simulink model "CCMHfaMotor"  (R2022b) (continued)
== system CCMHfaMotor == (continued)
  HfaActr05UrgcSts_HfaActr05UrgcSts/1 -> HfaMotor_Runnable_sys/171(HfaActr05UrgcSts_HfaActr05UrgcSts_read)
  HfaActr05VoltErr_HfaActr05VoltErr/1 -> HfaMotor_Runnable_sys/172(HfaActr05VoltErr_HfaActr05VoltErr_read)
  HfaActr06ActPson_HfaActr06ActPson/1 -> HfaMotor_Runnable_sys/173(HfaActr06ActPson_HfaActr06ActPson_read)
  HfaActr06ActPson_HfaActr06ActPson_IsUpdated/1 -> HfaMotor_Runnable_sys/174(HfaActr06ActPson_HfaActr06ActPson_IsUpdated_read)
  HfaActr06BlckInd_HfaActr06BlckInd/1 -> HfaMotor_Runnable_sys/175(HfaActr06BlckInd_HfaActr06BlckInd_read)
  HfaActr06BlckSts_HfaActr06BlckSts/1 -> HfaMotor_Runnable_sys/176(HfaActr06BlckSts_HfaActr06BlckSts_read)
  HfaActr06BusAdr_HfaActr06BusAdr/1 -> HfaMotor_Runnable_sys/177(HfaActr06BusAdr_HfaActr06BusAdr_read)
  HfaActr06CoilSts_HfaActr06CoilSts/1 -> HfaMotor_Runnable_sys/178(HfaActr06CoilSts_HfaActr06CoilSts_read)
  HfaActr06DrvDir_HfaActr06DrvDir/1 -> HfaMotor_Runnable_sys/179(HfaActr06DrvDir_HfaActr06DrvDir_read)
  HfaActr06ElecErr_HfaActr06ElecErr/1 -> HfaMotor_Runnable_sys/180(HfaActr06ElecErr_HfaActr06ElecErr_read)
  HfaActr06Mode_HfaActr06Mode/1 -> HfaMotor_Runnable_sys/181(HfaActr06Mode_HfaActr06Mode_read)
  HfaActr06MovDir_HfaActr06MovDir/1 -> HfaMotor_Runnable_sys/182(HfaActr06MovDir_HfaActr06MovDir_read)
  HfaActr06OvrTempErr_HfaActr06OvrTempErr/1 -> HfaMotor_Runnable_sys/183(HfaActr06OvrTempErr_HfaActr06OvrTempErr_read)
  HfaActr06PsonSts_HfaActr06PsonSts/1 -> HfaMotor_Runnable_sys/184(HfaActr06PsonSts_HfaActr06PsonSts_read)
  HfaActr06ResetSts_HfaActr06ResetSts/1 -> HfaMotor_Runnable_sys/185(HfaActr06ResetSts_HfaActr06ResetSts_read)
  HfaActr06SpclFct_HfaActr06SpclFct/1 -> HfaMotor_Runnable_sys/186(HfaActr06SpclFct_HfaActr06SpclFct_read)
  HfaActr06SpdSts_HfaActr06SpdSts/1 -> HfaMotor_Runnable_sys/187(HfaActr06SpdSts_HfaActr06SpdSts_read)
  HfaActr06TrqSts_HfaActr06TrqSts/1 -> HfaMotor_Runnable_sys/188(HfaActr06TrqSts_HfaActr06TrqSts_read)
  HfaActr06UrgcExist_HfaActr06UrgcExist/1 -> HfaMotor_Runnable_sys/189(HfaActr06UrgcExist_HfaActr06UrgcExist_read)
  HfaActr06UrgcPson_HfaActr06UrgcPson/1 -> HfaMotor_Runnable_sys/190(HfaActr06UrgcPson_HfaActr06UrgcPson_read)
  HfaActr06UrgcSts_HfaActr06UrgcSts/1 -> HfaMotor_Runnable_sys/191(HfaActr06UrgcSts_HfaActr06UrgcSts_read)
  HfaActr06VoltErr_HfaActr06VoltErr/1 -> HfaMotor_Runnable_sys/192(HfaActr06VoltErr_HfaActr06VoltErr_read)
  HfaActr07ActPson_HfaActr07ActPson/1 -> HfaMotor_Runnable_sys/193(HfaActr07ActPson_HfaActr07ActPson_read)
  HfaActr07ActPson_HfaActr07ActPson_IsUpdated/1 -> HfaMotor_Runnable_sys/194(HfaActr07ActPson_HfaActr07ActPson_IsUpdated_read)
  HfaActr07BlckInd_HfaActr07BlckInd/1 -> HfaMotor_Runnable_sys/195(HfaActr07BlckInd_HfaActr07BlckInd_read)
  HfaActr07BlckSts_HfaActr07BlckSts/1 -> HfaMotor_Runnable_sys/196(HfaActr07BlckSts_HfaActr07BlckSts_read)
  HfaActr07BusAdr_HfaActr07BusAdr/1 -> HfaMotor_Runnable_sys/197(HfaActr07BusAdr_HfaActr07BusAdr_read)
  HfaActr07CoilSts_HfaActr07CoilSts/1 -> HfaMotor_Runnable_sys/198(HfaActr07CoilSts_HfaActr07CoilSts_read)
  HfaActr07DrvDir_HfaActr07DrvDir/1 -> HfaMotor_Runnable_sys/199(HfaActr07DrvDir_HfaActr07DrvDir_read)
  HfaActr07ElecErr_HfaActr07ElecErr/1 -> HfaMotor_Runnable_sys/200(HfaActr07ElecErr_HfaActr07ElecErr_read)
  HfaActr07Mode_HfaActr07Mode/1 -> HfaMotor_Runnable_sys/201(HfaActr07Mode_HfaActr07Mode_read)
  HfaActr07MovDir_HfaActr07MovDir/1 -> HfaMotor_Runnable_sys/202(HfaActr07MovDir_HfaActr07MovDir_read)
  HfaActr07OvrTempErr_HfaActr07OvrTempErr/1 -> HfaMotor_Runnable_sys/203(HfaActr07OvrTempErr_HfaActr07OvrTempErr_read)
  HfaActr07PsonSts_HfaActr07PsonSts/1 -> HfaMotor_Runnable_sys/204(HfaActr07PsonSts_HfaActr07PsonSts_read)
  HfaActr07ResetSts_HfaActr07ResetSts/1 -> HfaMotor_Runnable_sys/205(HfaActr07ResetSts_HfaActr07ResetSts_read)
  HfaActr07SpclFct_HfaActr07SpclFct/1 -> HfaMotor_Runnable_sys/206(HfaActr07SpclFct_HfaActr07SpclFct_read)
  HfaActr07SpdSts_HfaActr07SpdSts/1 -> HfaMotor_Runnable_sys/207(HfaActr07SpdSts_HfaActr07SpdSts_read)
  HfaActr07TrqSts_HfaActr07TrqSts/1 -> HfaMotor_Runnable_sys/208(HfaActr07TrqSts_HfaActr07TrqSts_read)
  HfaActr07UrgcExist_HfaActr07UrgcExist/1 -> HfaMotor_Runnable_sys/209(HfaActr07UrgcExist_HfaActr07UrgcExist_read)
  HfaActr07UrgcPson_HfaActr07UrgcPson/1 -> HfaMotor_Runnable_sys/210(HfaActr07UrgcPson_HfaActr07UrgcPson_read)
  HfaActr07UrgcSts_HfaActr07UrgcSts/1 -> HfaMotor_Runnable_sys/211(HfaActr07UrgcSts_HfaActr07UrgcSts_read)
  HfaActr07VoltErr_HfaActr07VoltErr/1 -> HfaMotor_Runnable_sys/212(HfaActr07VoltErr_HfaActr07VoltErr_read)
  HfaActr08ActPson_HfaActr08ActPson/1 -> HfaMotor_Runnable_sys/213(HfaActr08ActPson_HfaActr08ActPson_read)
  HfaActr08ActPson_HfaActr08ActPson_IsUpdated/1 -> HfaMotor_Runnable_sys/214(HfaActr08ActPson_HfaActr08ActPson_IsUpdated_read)
  HfaActr08BlckInd_HfaActr08BlckInd/1 -> HfaMotor_Runnable_sys/215(HfaActr08BlckInd_HfaActr08BlckInd_read)
  HfaActr08BlckSts_HfaActr08BlckSts/1 -> HfaMotor_Runnable_sys/216(HfaActr08BlckSts_HfaActr08BlckSts_read)
  HfaActr08BusAdr_HfaActr08BusAdr/1 -> HfaMotor_Runnable_sys/217(HfaActr08BusAdr_HfaActr08BusAdr_read)
  HfaActr08CoilSts_HfaActr08CoilSts/1 -> HfaMotor_Runnable_sys/218(HfaActr08CoilSts_HfaActr08CoilSts_read)
  HfaActr08DrvDir_HfaActr08DrvDir/1 -> HfaMotor_Runnable_sys/219(HfaActr08DrvDir_HfaActr08DrvDir_read)
  HfaActr08ElecErr_HfaActr08ElecErr/1 -> HfaMotor_Runnable_sys/220(HfaActr08ElecErr_HfaActr08ElecErr_read)
  HfaActr08Mode_HfaActr08Mode/1 -> HfaMotor_Runnable_sys/221(HfaActr08Mode_HfaActr08Mode_read)
  HfaActr08MovDir_HfaActr08MovDir/1 -> HfaMotor_Runnable_sys/222(HfaActr08MovDir_HfaActr08MovDir_read)
  HfaActr08OvrTempErr_HfaActr08OvrTempErr/1 -> HfaMotor_Runnable_sys/223(HfaActr08OvrTempErr_HfaActr08OvrTempErr_read)
  HfaActr08PsonSts_HfaActr08PsonSts/1 -> HfaMotor_Runnable_sys/224(HfaActr08PsonSts_HfaActr08PsonSts_read)
  HfaActr08ResetSts_HfaActr08ResetSts/1 -> HfaMotor_Runnable_sys/225(HfaActr08ResetSts_HfaActr08ResetSts_read)
  HfaActr08SpclFct_HfaActr08SpclFct/1 -> HfaMotor_Runnable_sys/226(HfaActr08SpclFct_HfaActr08SpclFct_read)
  HfaActr08SpdSts_HfaActr08SpdSts/1 -> HfaMotor_Runnable_sys/227(HfaActr08SpdSts_HfaActr08SpdSts_read)
  HfaActr08TrqSts_HfaActr08TrqSts/1 -> HfaMotor_Runnable_sys/228(HfaActr08TrqSts_HfaActr08TrqSts_read)
  HfaActr08UrgcExist_HfaActr08UrgcExist/1 -> HfaMotor_Runnable_sys/229(HfaActr08UrgcExist_HfaActr08UrgcExist_read)
  HfaActr08UrgcPson_HfaActr08UrgcPson/1 -> HfaMotor_Runnable_sys/230(HfaActr08UrgcPson_HfaActr08UrgcPson_read)
  HfaActr08UrgcSts_HfaActr08UrgcSts/1 -> HfaMotor_Runnable_sys/231(HfaActr08UrgcSts_HfaActr08UrgcSts_read)
  HfaActr08VoltErr_HfaActr08VoltErr/1 -> HfaMotor_Runnable_sys/232(HfaActr08VoltErr_HfaActr08VoltErr_read)
  HfaActr09ActPson_HfaActr09ActPson/1 -> HfaMotor_Runnable_sys/233(HfaActr09ActPson_HfaActr09ActPson_read)
  HfaActr09ActPson_HfaActr09ActPson_IsUpdated/1 -> HfaMotor_Runnable_sys/234(HfaActr09ActPson_HfaActr09ActPson_IsUpdated_read)
  HfaActr09BlckInd_HfaActr09BlckInd/1 -> HfaMotor_Runnable_sys/235(HfaActr09BlckInd_HfaActr09BlckInd_read)
  HfaActr09BlckSts_HfaActr09BlckSts/1 -> HfaMotor_Runnable_sys/236(HfaActr09BlckSts_HfaActr09BlckSts_read)
  HfaActr09BusAdr_HfaActr09BusAdr/1 -> HfaMotor_Runnable_sys/237(HfaActr09BusAdr_HfaActr09BusAdr_read)
  HfaActr09CoilSts_HfaActr09CoilSts/1 -> HfaMotor_Runnable_sys/238(HfaActr09CoilSts_HfaActr09CoilSts_read)
  HfaActr09DrvDir_HfaActr09DrvDir/1 -> HfaMotor_Runnable_sys/239(HfaActr09DrvDir_HfaActr09DrvDir_read)
  HfaActr09ElecErr_HfaActr09ElecErr/1 -> HfaMotor_Runnable_sys/240(HfaActr09ElecErr_HfaActr09ElecErr_read)
  HfaActr09Mode_HfaActr09Mode/1 -> HfaMotor_Runnable_sys/241(HfaActr09Mode_HfaActr09Mode_read)
  HfaActr09MovDir_HfaActr09MovDir/1 -> HfaMotor_Runnable_sys/242(HfaActr09MovDir_HfaActr09MovDir_read)
  HfaActr09OvrTempErr_HfaActr09OvrTempErr/1 -> HfaMotor_Runnable_sys/243(HfaActr09OvrTempErr_HfaActr09OvrTempErr_read)
  HfaActr09PsonSts_HfaActr09PsonSts/1 -> HfaMotor_Runnable_sys/244(HfaActr09PsonSts_HfaActr09PsonSts_read)
  HfaActr09ResetSts_HfaActr09ResetSts/1 -> HfaMotor_Runnable_sys/245(HfaActr09ResetSts_HfaActr09ResetSts_read)
  HfaActr09SpclFct_HfaActr09SpclFct/1 -> HfaMotor_Runnable_sys/246(HfaActr09SpclFct_HfaActr09SpclFct_read)
  HfaActr09SpdSts_HfaActr09SpdSts/1 -> HfaMotor_Runnable_sys/247(HfaActr09SpdSts_HfaActr09SpdSts_read)
  HfaActr09TrqSts_HfaActr09TrqSts/1 -> HfaMotor_Runnable_sys/248(HfaActr09TrqSts_HfaActr09TrqSts_read)
  HfaActr09UrgcExist_HfaActr09UrgcExist/1 -> HfaMotor_Runnable_sys/249(HfaActr09UrgcExist_HfaActr09UrgcExist_read)
  HfaActr09UrgcPson_HfaActr09UrgcPson/1 -> HfaMotor_Runnable_sys/250(HfaActr09UrgcPson_HfaActr09UrgcPson_read)
  HfaActr09UrgcSts_HfaActr09UrgcSts/1 -> HfaMotor_Runnable_sys/251(HfaActr09UrgcSts_HfaActr09UrgcSts_read)
  HfaActr09VoltErr_HfaActr09VoltErr/1 -> HfaMotor_Runnable_sys/252(HfaActr09VoltErr_HfaActr09VoltErr_read)
  HfaActr10ActPson_HfaActr10ActPson/1 -> HfaMotor_Runnable_sys/253(HfaActr10ActPson_HfaActr10ActPson_read)
  HfaActr10ActPson_HfaActr10ActPson_IsUpdated/1 -> HfaMotor_Runnable_sys/254(HfaActr10ActPson_HfaActr10ActPson_IsUpdated_read)
  HfaActr10BlckInd_HfaActr10BlckInd/1 -> HfaMotor_Runnable_sys/255(HfaActr10BlckInd_HfaActr10BlckInd_read)
  HfaActr10BlckSts_HfaActr10BlckSts/1 -> HfaMotor_Runnable_sys/256(HfaActr10BlckSts_HfaActr10BlckSts_read)
  HfaActr10BusAdr_HfaActr10BusAdr/1 -> HfaMotor_Runnable_sys/257(HfaActr10BusAdr_HfaActr10BusAdr_read)
  HfaActr10CoilSts_HfaActr10CoilSts/1 -> HfaMotor_Runnable_sys/258(HfaActr10CoilSts_HfaActr10CoilSts_read)
  HfaActr10DrvDir_HfaActr10DrvDir/1 -> HfaMotor_Runnable_sys/259(HfaActr10DrvDir_HfaActr10DrvDir_read)
  HfaActr10ElecErr_HfaActr10ElecErr/1 -> HfaMotor_Runnable_sys/260(HfaActr10ElecErr_HfaActr10ElecErr_read)
  HfaActr10Mode_HfaActr10Mode/1 -> HfaMotor_Runnable_sys/261(HfaActr10Mode_HfaActr10Mode_read)
  HfaActr10MovDir_HfaActr10MovDir/1 -> HfaMotor_Runnable_sys/262(HfaActr10MovDir_HfaActr10MovDir_read)
  HfaActr10OvrTempErr_HfaActr10OvrTempErr/1 -> HfaMotor_Runnable_sys/263(HfaActr10OvrTempErr_HfaActr10OvrTempErr_read)
  HfaActr10PsonSts_HfaActr10PsonSts/1 -> HfaMotor_Runnable_sys/264(HfaActr10PsonSts_HfaActr10PsonSts_read)
  HfaActr10ResetSts_HfaActr10ResetSts/1 -> HfaMotor_Runnable_sys/265(HfaActr10ResetSts_HfaActr10ResetSts_read)
  HfaActr10SpclFct_HfaActr10SpclFct/1 -> HfaMotor_Runnable_sys/266(HfaActr10SpclFct_HfaActr10SpclFct_read)
  HfaActr10SpdSts_HfaActr10SpdSts/1 -> HfaMotor_Runnable_sys/267(HfaActr10SpdSts_HfaActr10SpdSts_read)
  HfaActr10TrqSts_HfaActr10TrqSts/1 -> HfaMotor_Runnable_sys/268(HfaActr10TrqSts_HfaActr10TrqSts_read)
  HfaActr10UrgcExist_HfaActr10UrgcExist/1 -> HfaMotor_Runnable_sys/269(HfaActr10UrgcExist_HfaActr10UrgcExist_read)
  HfaActr10UrgcPson_HfaActr10UrgcPson/1 -> HfaMotor_Runnable_sys/270(HfaActr10UrgcPson_HfaActr10UrgcPson_read)
  HfaActr10UrgcSts_HfaActr10UrgcSts/1 -> HfaMotor_Runnable_sys/271(HfaActr10UrgcSts_HfaActr10UrgcSts_read)
  HfaActr10VoltErr_HfaActr10VoltErr/1 -> HfaMotor_Runnable_sys/272(HfaActr10VoltErr_HfaActr10VoltErr_read)
  HfaActr11ActPson_HfaActr11ActPson/1 -> HfaMotor_Runnable_sys/273(HfaActr11ActPson_HfaActr11ActPson_read)
  HfaActr11ActPson_HfaActr11ActPson_IsUpdated/1 -> HfaMotor_Runnable_sys/274(HfaActr11ActPson_HfaActr11ActPson_IsUpdated_read)
  HfaActr11BlckInd_HfaActr11BlckInd/1 -> HfaMotor_Runnable_sys/275(HfaActr11BlckInd_HfaActr11BlckInd_read)
  HfaActr11BlckSts_HfaActr11BlckSts/1 -> HfaMotor_Runnable_sys/276(HfaActr11BlckSts_HfaActr11BlckSts_read)
  HfaActr11BusAdr_HfaActr11BusAdr/1 -> HfaMotor_Runnable_sys/277(HfaActr11BusAdr_HfaActr11BusAdr_read)
  HfaActr11CoilSts_HfaActr11CoilSts/1 -> HfaMotor_Runnable_sys/278(HfaActr11CoilSts_HfaActr11CoilSts_read)
  HfaActr11DrvDir_HfaActr11DrvDir/1 -> HfaMotor_Runnable_sys/279(HfaActr11DrvDir_HfaActr11DrvDir_read)
  HfaActr11ElecErr_HfaActr11ElecErr/1 -> HfaMotor_Runnable_sys/280(HfaActr11ElecErr_HfaActr11ElecErr_read)
  HfaActr11Mode_HfaActr11Mode/1 -> HfaMotor_Runnable_sys/281(HfaActr11Mode_HfaActr11Mode_read)
  HfaActr11MovDir_HfaActr11MovDir/1 -> HfaMotor_Runnable_sys/282(HfaActr11MovDir_HfaActr11MovDir_read)
  HfaActr11OvrTempErr_HfaActr11OvrTempErr/1 -> HfaMotor_Runnable_sys/283(HfaActr11OvrTempErr_HfaActr11OvrTempErr_read)
  HfaActr11PsonSts_HfaActr11PsonSts/1 -> HfaMotor_Runnable_sys/284(HfaActr11PsonSts_HfaActr11PsonSts_read)
  HfaActr11ResetSts_HfaActr11ResetSts/1 -> HfaMotor_Runnable_sys/285(HfaActr11ResetSts_HfaActr11ResetSts_read)
  HfaActr11SpclFct_HfaActr11SpclFct/1 -> HfaMotor_Runnable_sys/286(HfaActr11SpclFct_HfaActr11SpclFct_read)
  HfaActr11SpdSts_HfaActr11SpdSts/1 -> HfaMotor_Runnable_sys/287(HfaActr11SpdSts_HfaActr11SpdSts_read)
  HfaActr11TrqSts_HfaActr11TrqSts/1 -> HfaMotor_Runnable_sys/288(HfaActr11TrqSts_HfaActr11TrqSts_read)
  HfaActr11UrgcExist_HfaActr11UrgcExist/1 -> HfaMotor_Runnable_sys/289(HfaActr11UrgcExist_HfaActr11UrgcExist_read)
  HfaActr11UrgcPson_HfaActr11UrgcPson/1 -> HfaMotor_Runnable_sys/290(HfaActr11UrgcPson_HfaActr11UrgcPson_read)
  HfaActr11UrgcSts_HfaActr11UrgcSts/1 -> HfaMotor_Runnable_sys/291(HfaActr11UrgcSts_HfaActr11UrgcSts_read)
  HfaActr11VoltErr_HfaActr11VoltErr/1 -> HfaMotor_Runnable_sys/292(HfaActr11VoltErr_HfaActr11VoltErr_read)
  HfaActr12ActPson_HfaActr12ActPson/1 -> HfaMotor_Runnable_sys/293(HfaActr12ActPson_HfaActr12ActPson_read)
  HfaActr12ActPson_HfaActr12ActPson_IsUpdated/1 -> HfaMotor_Runnable_sys/294(HfaActr12ActPson_HfaActr12ActPson_IsUpdated_read)
  HfaActr12BlckInd_HfaActr12BlckInd/1 -> HfaMotor_Runnable_sys/295(HfaActr12BlckInd_HfaActr12BlckInd_read)
  HfaActr12BlckSts_HfaActr12BlckSts/1 -> HfaMotor_Runnable_sys/296(HfaActr12BlckSts_HfaActr12BlckSts_read)
  HfaActr12BusAdr_HfaActr12BusAdr/1 -> HfaMotor_Runnable_sys/297(HfaActr12BusAdr_HfaActr12BusAdr_read)
  HfaActr12CoilSts_HfaActr12CoilSts/1 -> HfaMotor_Runnable_sys/298(HfaActr12CoilSts_HfaActr12CoilSts_read)
  HfaActr12DrvDir_HfaActr12DrvDir/1 -> HfaMotor_Runnable_sys/299(HfaActr12DrvDir_HfaActr12DrvDir_read)
  HfaActr12ElecErr_HfaActr12ElecErr/1 -> HfaMotor_Runnable_sys/300(HfaActr12ElecErr_HfaActr12ElecErr_read)
  HfaActr12Mode_HfaActr12Mode/1 -> HfaMotor_Runnable_sys/301(HfaActr12Mode_HfaActr12Mode_read)
  HfaActr12MovDir_HfaActr12MovDir/1 -> HfaMotor_Runnable_sys/302(HfaActr12MovDir_HfaActr12MovDir_read)
  HfaActr12OvrTempErr_HfaActr12OvrTempErr/1 -> HfaMotor_Runnable_sys/303(HfaActr12OvrTempErr_HfaActr12OvrTempErr_read)
  HfaActr12PsonSts_HfaActr12PsonSts/1 -> HfaMotor_Runnable_sys/304(HfaActr12PsonSts_HfaActr12PsonSts_read)
  HfaActr12ResetSts_HfaActr12ResetSts/1 -> HfaMotor_Runnable_sys/305(HfaActr12ResetSts_HfaActr12ResetSts_read)
  HfaActr12SpclFct_HfaActr12SpclFct/1 -> HfaMotor_Runnable_sys/306(HfaActr12SpclFct_HfaActr12SpclFct_read)
  HfaActr12SpdSts_HfaActr12SpdSts/1 -> HfaMotor_Runnable_sys/307(HfaActr12SpdSts_HfaActr12SpdSts_read)
  HfaActr12TrqSts_HfaActr12TrqSts/1 -> HfaMotor_Runnable_sys/308(HfaActr12TrqSts_HfaActr12TrqSts_read)
  HfaActr12UrgcExist_HfaActr12UrgcExist/1 -> HfaMotor_Runnable_sys/309(HfaActr12UrgcExist_HfaActr12UrgcExist_read)
  HfaActr12UrgcPson_HfaActr12UrgcPson/1 -> HfaMotor_Runnable_sys/310(HfaActr12UrgcPson_HfaActr12UrgcPson_read)
  HfaActr12UrgcSts_HfaActr12UrgcSts/1 -> HfaMotor_Runnable_sys/311(HfaActr12UrgcSts_HfaActr12UrgcSts_read)
  HfaActr12VoltErr_HfaActr12VoltErr/1 -> HfaMotor_Runnable_sys/312(HfaActr12VoltErr_HfaActr12VoltErr_read)
  HfaActr13ActPson_HfaActr13ActPson/1 -> HfaMotor_Runnable_sys/313(HfaActr13ActPson_HfaActr13ActPson_read)
  HfaActr13ActPson_HfaActr13ActPson_IsUpdated/1 -> HfaMotor_Runnable_sys/314(HfaActr13ActPson_HfaActr13ActPson_IsUpdated_read)
  HfaActr13BlckInd_HfaActr13BlckInd/1 -> HfaMotor_Runnable_sys/315(HfaActr13BlckInd_HfaActr13BlckInd_read)
  HfaActr13BlckSts_HfaActr13BlckSts/1 -> HfaMotor_Runnable_sys/316(HfaActr13BlckSts_HfaActr13BlckSts_read)
  HfaActr13BusAdr_HfaActr13BusAdr/1 -> HfaMotor_Runnable_sys/317(HfaActr13BusAdr_HfaActr13BusAdr_read)
  HfaActr13CoilSts_HfaActr13CoilSts/1 -> HfaMotor_Runnable_sys/318(HfaActr13CoilSts_HfaActr13CoilSts_read)
  HfaActr13DrvDir_HfaActr13DrvDir/1 -> HfaMotor_Runnable_sys/319(HfaActr13DrvDir_HfaActr13DrvDir_read)
  HfaActr13ElecErr_HfaActr13ElecErr/1 -> HfaMotor_Runnable_sys/320(HfaActr13ElecErr_HfaActr13ElecErr_read)
  HfaActr13Mode_HfaActr13Mode/1 -> HfaMotor_Runnable_sys/321(HfaActr13Mode_HfaActr13Mode_read)
  HfaActr13MovDir_HfaActr13MovDir/1 -> HfaMotor_Runnable_sys/322(HfaActr13MovDir_HfaActr13MovDir_read)
  HfaActr13OvrTempErr_HfaActr13OvrTempErr/1 -> HfaMotor_Runnable_sys/323(HfaActr13OvrTempErr_HfaActr13OvrTempErr_read)
  HfaActr13PsonSts_HfaActr13PsonSts/1 -> HfaMotor_Runnable_sys/324(HfaActr13PsonSts_HfaActr13PsonSts_read)
  HfaActr13ResetSts_HfaActr13ResetSts/1 -> HfaMotor_Runnable_sys/325(HfaActr13ResetSts_HfaActr13ResetSts_read)
  HfaActr13SpclFct_HfaActr13SpclFct/1 -> HfaMotor_Runnable_sys/326(HfaActr13SpclFct_HfaActr13SpclFct_read)
  HfaActr13SpdSts_HfaActr13SpdSts/1 -> HfaMotor_Runnable_sys/327(HfaActr13SpdSts_HfaActr13SpdSts_read)
  HfaActr13TrqSts_HfaActr13TrqSts/1 -> HfaMotor_Runnable_sys/328(HfaActr13TrqSts_HfaActr13TrqSts_read)
  HfaActr13UrgcExist_HfaActr13UrgcExist/1 -> HfaMotor_Runnable_sys/329(HfaActr13UrgcExist_HfaActr13UrgcExist_read)
  HfaActr13UrgcPson_HfaActr13UrgcPson/1 -> HfaMotor_Runnable_sys/330(HfaActr13UrgcPson_HfaActr13UrgcPson_read)
  HfaActr13UrgcSts_HfaActr13UrgcSts/1 -> HfaMotor_Runnable_sys/331(HfaActr13UrgcSts_HfaActr13UrgcSts_read)
  HfaActr13VoltErr_HfaActr13VoltErr/1 -> HfaMotor_Runnable_sys/332(HfaActr13VoltErr_HfaActr13VoltErr_read)
  HfaActr14ActPson_HfaActr14ActPson/1 -> HfaMotor_Runnable_sys/333(HfaActr14ActPson_HfaActr14ActPson_read)
  HfaActr14ActPson_HfaActr14ActPson_IsUpdated/1 -> HfaMotor_Runnable_sys/334(HfaActr14ActPson_HfaActr14ActPson_IsUpdated_read)
  HfaActr14BlckInd_HfaActr14BlckInd/1 -> HfaMotor_Runnable_sys/335(HfaActr14BlckInd_HfaActr14BlckInd_read)
  HfaActr14BlckSts_HfaActr14BlckSts/1 -> HfaMotor_Runnable_sys/336(HfaActr14BlckSts_HfaActr14BlckSts_read)
  HfaActr14BusAdr_HfaActr14BusAdr/1 -> HfaMotor_Runnable_sys/337(HfaActr14BusAdr_HfaActr14BusAdr_read)
  HfaActr14CoilSts_HfaActr14CoilSts/1 -> HfaMotor_Runnable_sys/338(HfaActr14CoilSts_HfaActr14CoilSts_read)
  HfaActr14DrvDir_HfaActr14DrvDir/1 -> HfaMotor_Runnable_sys/339(HfaActr14DrvDir_HfaActr14DrvDir_read)
  HfaActr14ElecErr_HfaActr14ElecErr/1 -> HfaMotor_Runnable_sys/340(HfaActr14ElecErr_HfaActr14ElecErr_read)
  HfaActr14Mode_HfaActr14Mode/1 -> HfaMotor_Runnable_sys/341(HfaActr14Mode_HfaActr14Mode_read)
  HfaActr14MovDir_HfaActr14MovDir/1 -> HfaMotor_Runnable_sys/342(HfaActr14MovDir_HfaActr14MovDir_read)
  HfaActr14OvrTempErr_HfaActr14OvrTempErr/1 -> HfaMotor_Runnable_sys/343(HfaActr14OvrTempErr_HfaActr14OvrTempErr_read)
  HfaActr14PsonSts_HfaActr14PsonSts/1 -> HfaMotor_Runnable_sys/344(HfaActr14PsonSts_HfaActr14PsonSts_read)
  HfaActr14ResetSts_HfaActr14ResetSts/1 -> HfaMotor_Runnable_sys/345(HfaActr14ResetSts_HfaActr14ResetSts_read)
  HfaActr14SpclFct_HfaActr14SpclFct/1 -> HfaMotor_Runnable_sys/346(HfaActr14SpclFct_HfaActr14SpclFct_read)
  HfaActr14SpdSts_HfaActr14SpdSts/1 -> HfaMotor_Runnable_sys/347(HfaActr14SpdSts_HfaActr14SpdSts_read)
  HfaActr14TrqSts_HfaActr14TrqSts/1 -> HfaMotor_Runnable_sys/348(HfaActr14TrqSts_HfaActr14TrqSts_read)
  HfaActr14UrgcExist_HfaActr14UrgcExist/1 -> HfaMotor_Runnable_sys/349(HfaActr14UrgcExist_HfaActr14UrgcExist_read)
  HfaActr14UrgcPson_HfaActr14UrgcPson/1 -> HfaMotor_Runnable_sys/350(HfaActr14UrgcPson_HfaActr14UrgcPson_read)
  HfaActr14UrgcSts_HfaActr14UrgcSts/1 -> HfaMotor_Runnable_sys/351(HfaActr14UrgcSts_HfaActr14UrgcSts_read)
  HfaActr14VoltErr_HfaActr14VoltErr/1 -> HfaMotor_Runnable_sys/352(HfaActr14VoltErr_HfaActr14VoltErr_read)
  HfaActr15ActPson_HfaActr15ActPson/1 -> HfaMotor_Runnable_sys/353(HfaActr15ActPson_HfaActr15ActPson_read)
  HfaActr15ActPson_HfaActr15ActPson_IsUpdated/1 -> HfaMotor_Runnable_sys/354(HfaActr15ActPson_HfaActr15ActPson_IsUpdated_read)
  HfaActr15BlckInd_HfaActr15BlckInd/1 -> HfaMotor_Runnable_sys/355(HfaActr15BlckInd_HfaActr15BlckInd_read)
  HfaActr15BlckSts_HfaActr15BlckSts/1 -> HfaMotor_Runnable_sys/356(HfaActr15BlckSts_HfaActr15BlckSts_read)
  HfaActr15BusAdr_HfaActr15BusAdr/1 -> HfaMotor_Runnable_sys/357(HfaActr15BusAdr_HfaActr15BusAdr_read)
  HfaActr15CoilSts_HfaActr15CoilSts/1 -> HfaMotor_Runnable_sys/358(HfaActr15CoilSts_HfaActr15CoilSts_read)
  HfaActr15DrvDir_HfaActr15DrvDir/1 -> HfaMotor_Runnable_sys/359(HfaActr15DrvDir_HfaActr15DrvDir_read)
  HfaActr15ElecErr_HfaActr15ElecErr/1 -> HfaMotor_Runnable_sys/360(HfaActr15ElecErr_HfaActr15ElecErr_read)
  HfaActr15Mode_HfaActr15Mode/1 -> HfaMotor_Runnable_sys/361(HfaActr15Mode_HfaActr15Mode_read)
  HfaActr15MovDir_HfaActr15MovDir/1 -> HfaMotor_Runnable_sys/362(HfaActr15MovDir_HfaActr15MovDir_read)
  HfaActr15OvrTempErr_HfaActr15OvrTempErr/1 -> HfaMotor_Runnable_sys/363(HfaActr15OvrTempErr_HfaActr15OvrTempErr_read)
  HfaActr15PsonSts_HfaActr15PsonSts/1 -> HfaMotor_Runnable_sys/364(HfaActr15PsonSts_HfaActr15PsonSts_read)
  HfaActr15ResetSts_HfaActr15ResetSts/1 -> HfaMotor_Runnable_sys/365(HfaActr15ResetSts_HfaActr15ResetSts_read)
  HfaActr15SpclFct_HfaActr15SpclFct/1 -> HfaMotor_Runnable_sys/366(HfaActr15SpclFct_HfaActr15SpclFct_read)
  HfaActr15SpdSts_HfaActr15SpdSts/1 -> HfaMotor_Runnable_sys/367(HfaActr15SpdSts_HfaActr15SpdSts_read)
  HfaActr15TrqSts_HfaActr15TrqSts/1 -> HfaMotor_Runnable_sys/368(HfaActr15TrqSts_HfaActr15TrqSts_read)
  HfaActr15UrgcExist_HfaActr15UrgcExist/1 -> HfaMotor_Runnable_sys/369(HfaActr15UrgcExist_HfaActr15UrgcExist_read)
  HfaActr15UrgcPson_HfaActr15UrgcPson/1 -> HfaMotor_Runnable_sys/370(HfaActr15UrgcPson_HfaActr15UrgcPson_read)
  HfaActr15UrgcSts_HfaActr15UrgcSts/1 -> HfaMotor_Runnable_sys/371(HfaActr15UrgcSts_HfaActr15UrgcSts_read)
  HfaActr15VoltErr_HfaActr15VoltErr/1 -> HfaMotor_Runnable_sys/372(HfaActr15VoltErr_HfaActr15VoltErr_read)
  HvacCfgForClimaCtrl_HvacCfgForAQSTypes/1 -> HfaMotor_Runnable_sys/373(HvacCfgForClimaCtrl_HvacCfgForAQSTypes_read)
  HvacCfgForClimaCtrl_HvacCfgForHvacLowSideRETXVESts/1 -> HfaMotor_Runnable_sys/374(HvacCfgForClimaCtrl_HvacCfgForHvacLowSideRETXVESts_read)
  HvacCfgForClimaCtrl_HvacCfgForHvacReBlowerType/1 -> HfaMotor_Runnable_sys/375(HvacCfgForClimaCtrl_HvacCfgForHvacReBlowerType_read)
  HvacCfgForClimaCtrl_HvacCfgForHvacReVentPstn/1 -> HfaMotor_Runnable_sys/376(HvacCfgForClimaCtrl_HvacCfgForHvacReVentPstn_read)
  HvacCfgForClimaCtrl_HvacCfgForHvacSnsrRCmptmtESts/1 -> HfaMotor_Runnable_sys/377(HvacCfgForClimaCtrl_HvacCfgForHvacSnsrRCmptmtESts_read)
  HvacCfgForClimaCtrl_HvacCfgForHvacSnsrReHexESts/1 -> HfaMotor_Runnable_sys/378(HvacCfgForClimaCtrl_HvacCfgForHvacSnsrReHexESts_read)
  Lin1ActvReady_Lin1ActvReady/1 -> HfaMotor_Runnable_sys/379(Lin1ActvReady_Lin1ActvReady_read)
  Lin2ActvReady_Lin2ActvReady/1 -> HfaMotor_Runnable_sys/380(Lin2ActvReady_Lin2ActvReady_read)
  ReHvacActrActPson_ReHvacActrActPson/1 -> HfaMotor_Runnable_sys/381(ReHvacActrActPson_ReHvacActrActPson_read)
  ReHvacActrActPson_ReHvacActrActPson_IsUpdated/1 -> HfaMotor_Runnable_sys/382(ReHvacActrActPson_ReHvacActrActPson_IsUpdated_read)
  ReHvacActrBlckInd_ReHvacActrBlckInd/1 -> HfaMotor_Runnable_sys/383(ReHvacActrBlckInd_ReHvacActrBlckInd_read)
  ReHvacActrBlckSts_ReHvacActrBlckSts/1 -> HfaMotor_Runnable_sys/384(ReHvacActrBlckSts_ReHvacActrBlckSts_read)
  ReHvacActrBusAdr_ReHvacActrBusAdr/1 -> HfaMotor_Runnable_sys/385(ReHvacActrBusAdr_ReHvacActrBusAdr_read)
  ReHvacActrCoilSts_ReHvacActrCoilSts/1 -> HfaMotor_Runnable_sys/386(ReHvacActrCoilSts_ReHvacActrCoilSts_read)
  ReHvacActrDrvDir_ReHvacActrDrvDir/1 -> HfaMotor_Runnable_sys/387(ReHvacActrDrvDir_ReHvacActrDrvDir_read)
  ReHvacActrElecErr_ReHvacActrElecErr/1 -> HfaMotor_Runnable_sys/388(ReHvacActrElecErr_ReHvacActrElecErr_read)
  ReHvacActrMode_ReHvacActrMode/1 -> HfaMotor_Runnable_sys/389(ReHvacActrMode_ReHvacActrMode_read)
  ReHvacActrMovDir_ReHvacActrMovDir/1 -> HfaMotor_Runnable_sys/390(ReHvacActrMovDir_ReHvacActrMovDir_read)
  ReHvacActrOvrTempErr_ReHvacActrOvrTempErr/1 -> HfaMotor_Runnable_sys/391(ReHvacActrOvrTempErr_ReHvacActrOvrTempErr_read)
  ReHvacActrPsonSts_ReHvacActrPsonSts/1 -> HfaMotor_Runnable_sys/392(ReHvacActrPsonSts_ReHvacActrPsonSts_read)
  ReHvacActrResetSts_ReHvacActrResetSts/1 -> HfaMotor_Runnable_sys/393(ReHvacActrResetSts_ReHvacActrResetSts_read)
  ReHvacActrSpclFct_ReHvacActrSpclFct/1 -> HfaMotor_Runnable_sys/394(ReHvacActrSpclFct_ReHvacActrSpclFct_read)
  ReHvacActrSpdSts_ReHvacActrSpdSts/1 -> HfaMotor_Runnable_sys/395(ReHvacActrSpdSts_ReHvacActrSpdSts_read)
  ReHvacActrTrqSts_ReHvacActrTrqSts/1 -> HfaMotor_Runnable_sys/396(ReHvacActrTrqSts_ReHvacActrTrqSts_read)
  ReHvacActrUrgcExist_ReHvacActrUrgcExist/1 -> HfaMotor_Runnable_sys/397(ReHvacActrUrgcExist_ReHvacActrUrgcExist_read)
  ReHvacActrUrgcPson_ReHvacActrUrgcPson/1 -> HfaMotor_Runnable_sys/398(ReHvacActrUrgcPson_ReHvacActrUrgcPson_read)
  ReHvacActrUrgcSts_ReHvacActrUrgcSts/1 -> HfaMotor_Runnable_sys/399(ReHvacActrUrgcSts_ReHvacActrUrgcSts_read)
  ReHvacActrVoltErr_ReHvacActrVoltErr/1 -> HfaMotor_Runnable_sys/400(ReHvacActrVoltErr_ReHvacActrVoltErr_read)
  SysServCarCfgSts_SysServCCPBGMNum/1 -> HfaMotor_Runnable_sys/401(SysServCarCfgSts_SysServCCPBGMNum_read)
  SysServCarCfgSts_SysServCCPLin2AQSSts/1 -> HfaMotor_Runnable_sys/402(SysServCarCfgSts_SysServCCPLin2AQSSts_read)
  SysServCarCfgSts_SysServDUBlowerPwmSignTypeSts/1 -> HfaMotor_Runnable_sys/403(SysServCarCfgSts_SysServDUBlowerPwmSignTypeSts_read)
  SysServCarCfgSts_SysServDUDCFLMdeSts/1 -> HfaMotor_Runnable_sys/404(SysServCarCfgSts_SysServDUDCFLMdeSts_read)
  SysServCarCfgSts_SysServDULin4HFAFStsH/1 -> HfaMotor_Runnable_sys/405(SysServCarCfgSts_SysServDULin4HFAFStsH_read)
  SysServCarCfgSts_SysServDUReBlowerPwmSignSts/1 -> HfaMotor_Runnable_sys/406(SysServCarCfgSts_SysServDUReBlowerPwmSignSts_read)
  SysServCarCfgSts_SysServDUReBlowerPwmSts/1 -> HfaMotor_Runnable_sys/407(SysServCarCfgSts_SysServDUReBlowerPwmSts_read)
  SysServCarCfgSts_SysServFloatingMotorSts/1 -> HfaMotor_Runnable_sys/408(SysServCarCfgSts_SysServFloatingMotorSts_read)
  SysServCarCfgSts_SysServFrntHEXSnsrPosn/1 -> HfaMotor_Runnable_sys/409(SysServCarCfgSts_SysServFrntHEXSnsrPosn_read)
  SysServCarCfgSts_SysServLowSideRETXVSts/1 -> HfaMotor_Runnable_sys/410(SysServCarCfgSts_SysServLowSideRETXVSts_read)
  SysServCarCfgSts_SysServReHEXSnsrPosn/1 -> HfaMotor_Runnable_sys/411(SysServCarCfgSts_SysServReHEXSnsrPosn_read)
  SysServCarCfgSts_SysServRHFASts/1 -> HfaMotor_Runnable_sys/412(SysServCarCfgSts_SysServRHFASts_read)
  SysServCarCfgSts_SysServSnsrForFCmptmtTType/1 -> HfaMotor_Runnable_sys/413(SysServCarCfgSts_SysServSnsrForFCmptmtTType_read)
  WtrActrLeActPson_WtrActrLeActPson/1 -> HfaMotor_Runnable_sys/414(WtrActrLeActPson_WtrActrLeActPson_read)
  WtrActrLeBlckInd_WtrActrLeBlckInd/1 -> HfaMotor_Runnable_sys/415(WtrActrLeBlckInd_WtrActrLeBlckInd_read)
  WtrActrLeBlckSts_WtrActrLeBlckSts/1 -> HfaMotor_Runnable_sys/416(WtrActrLeBlckSts_WtrActrLeBlckSts_read)
  WtrActrLeBusAdr_WtrActrLeBusAdr/1 -> HfaMotor_Runnable_sys/417(WtrActrLeBusAdr_WtrActrLeBusAdr_read)
  WtrActrLeCoilSts_WtrActrLeCoilSts/1 -> HfaMotor_Runnable_sys/418(WtrActrLeCoilSts_WtrActrLeCoilSts_read)
  WtrActrLeDir_WtrActrLeDir/1 -> HfaMotor_Runnable_sys/419(WtrActrLeDir_WtrActrLeDir_read)
  WtrActrLeElecErr_WtrActrLeElecErr/1 -> HfaMotor_Runnable_sys/420(WtrActrLeElecErr_WtrActrLeElecErr_read)
  WtrActrLeMode_WtrActrLeMode/1 -> HfaMotor_Runnable_sys/421(WtrActrLeMode_WtrActrLeMode_read)
  WtrActrLeMovDir_WtrActrLeMovDir/1 -> HfaMotor_Runnable_sys/422(WtrActrLeMovDir_WtrActrLeMovDir_read)
  WtrActrLeOvrTempErr_WtrActrLeOvrTempErr/1 -> HfaMotor_Runnable_sys/423(WtrActrLeOvrTempErr_WtrActrLeOvrTempErr_read)
  WtrActrLePsonSts_WtrActrLePsonSts/1 -> HfaMotor_Runnable_sys/424(WtrActrLePsonSts_WtrActrLePsonSts_read)
  WtrActrLeResetSts_WtrActrLeResetSts/1 -> HfaMotor_Runnable_sys/425(WtrActrLeResetSts_WtrActrLeResetSts_read)
  WtrActrLeSpclFct_WtrActrLeSpclFct/1 -> HfaMotor_Runnable_sys/426(WtrActrLeSpclFct_WtrActrLeSpclFct_read)
  WtrActrLeSpdSts_WtrActrLeSpdSts/1 -> HfaMotor_Runnable_sys/427(WtrActrLeSpdSts_WtrActrLeSpdSts_read)
  WtrActrLeTrqSts_WtrActrLeTrqSts/1 -> HfaMotor_Runnable_sys/428(WtrActrLeTrqSts_WtrActrLeTrqSts_read)
  WtrActrLeUrgcExist_WtrActrLeUrgcExist/1 -> HfaMotor_Runnable_sys/429(WtrActrLeUrgcExist_WtrActrLeUrgcExist_read)
  WtrActrLeUrgcPson_WtrActrLeUrgcPson/1 -> HfaMotor_Runnable_sys/430(WtrActrLeUrgcPson_WtrActrLeUrgcPson_read)
  WtrActrLeUrgcSts_WtrActrLeUrgcSts/1 -> HfaMotor_Runnable_sys/431(WtrActrLeUrgcSts_WtrActrLeUrgcSts_read)
  WtrActrLeVoltErr_WtrActrLeVoltErr/1 -> HfaMotor_Runnable_sys/432(WtrActrLeVoltErr_WtrActrLeVoltErr_read)
  WtrActrRiActPson_WtrActrRiActPson/1 -> HfaMotor_Runnable_sys/433(WtrActrRiActPson_WtrActrRiActPson_read)
  WtrActrRiBlckInd_WtrActrRiBlckInd/1 -> HfaMotor_Runnable_sys/434(WtrActrRiBlckInd_WtrActrRiBlckInd_read)
  WtrActrRiBlckSts_WtrActrRiBlckSts/1 -> HfaMotor_Runnable_sys/435(WtrActrRiBlckSts_WtrActrRiBlckSts_read)
  WtrActrRiBusAdr_WtrActrRiBusAdr/1 -> HfaMotor_Runnable_sys/436(WtrActrRiBusAdr_WtrActrRiBusAdr_read)
  WtrActrRiCoilSts_WtrActrRiCoilSts/1 -> HfaMotor_Runnable_sys/437(WtrActrRiCoilSts_WtrActrRiCoilSts_read)
  WtrActrRiDir_WtrActrRiDir/1 -> HfaMotor_Runnable_sys/438(WtrActrRiDir_WtrActrRiDir_read)
  WtrActrRiElecErr_WtrActrRiElecErr/1 -> HfaMotor_Runnable_sys/439(WtrActrRiElecErr_WtrActrRiElecErr_read)
  WtrActrRiMode_WtrActrRiMode/1 -> HfaMotor_Runnable_sys/440(WtrActrRiMode_WtrActrRiMode_read)
  WtrActrRiMovDir_WtrActrRiMovDir/1 -> HfaMotor_Runnable_sys/441(WtrActrRiMovDir_WtrActrRiMovDir_read)
  WtrActrRiOvrTempErr_WtrActrRiOvrTempErr/1 -> HfaMotor_Runnable_sys/442(WtrActrRiOvrTempErr_WtrActrRiOvrTempErr_read)
  WtrActrRiPsonSts_WtrActrRiPsonSts/1 -> HfaMotor_Runnable_sys/443(WtrActrRiPsonSts_WtrActrRiPsonSts_read)
  WtrActrRiResetSts_WtrActrRiResetSts/1 -> HfaMotor_Runnable_sys/444(WtrActrRiResetSts_WtrActrRiResetSts_read)
  WtrActrRiSpclFct_WtrActrRiSpclFct/1 -> HfaMotor_Runnable_sys/445(WtrActrRiSpclFct_WtrActrRiSpclFct_read)
  WtrActrRiSpdSts_WtrActrRiSpdSts/1 -> HfaMotor_Runnable_sys/446(WtrActrRiSpdSts_WtrActrRiSpdSts_read)
  WtrActrRiTrqSts_WtrActrRiTrqSts/1 -> HfaMotor_Runnable_sys/447(WtrActrRiTrqSts_WtrActrRiTrqSts_read)
  WtrActrRiUrgcExist_WtrActrRiUrgcExist/1 -> HfaMotor_Runnable_sys/448(WtrActrRiUrgcExist_WtrActrRiUrgcExist_read)
  WtrActrRiUrgcPson_WtrActrRiUrgcPson/1 -> HfaMotor_Runnable_sys/449(WtrActrRiUrgcPson_WtrActrRiUrgcPson_read)
  WtrActrRiUrgcSts_WtrActrRiUrgcSts/1 -> HfaMotor_Runnable_sys/450(WtrActrRiUrgcSts_WtrActrRiUrgcSts_read)
  WtrActrRiVoltErr_WtrActrRiVoltErr/1 -> HfaMotor_Runnable_sys/451(WtrActrRiVoltErr_WtrActrRiVoltErr_read)
  HfaMotor_Runnable_sys/1(ActCfrAddrFinalAddr_ActCfrAddrFinalAddr_write) -> ActCfrAddrFinalAddr_ActCfrAddrFinalAddr/1
  HfaMotor_Runnable_sys/2(ActCfrAddrGroup_ActCfrAddrGroup_write) -> ActCfrAddrGroup_ActCfrAddrGroup/1
  HfaMotor_Runnable_sys/3(ActCfrAddrItem_ActCfrAddrItem_write) -> ActCfrAddrItem_ActCfrAddrItem/1
  HfaMotor_Runnable_sys/4(ActCfrIniCPos_ActCfrIniCPos_write) -> ActCfrIniCPos_ActCfrIniCPos/1
  HfaMotor_Runnable_sys/5(ActCfrIniGroup_ActCfrIniGroup_write) -> ActCfrIniGroup_ActCfrIniGroup/1
  HfaMotor_Runnable_sys/6(ActCfrIniItem_ActCfrIniItem_write) -> ActCfrIniItem_ActCfrIniItem/1
  HfaMotor_Runnable_sys/7(ActCfrIniResvd_ActCfrIniResvd_write) -> ActCfrIniResvd_ActCfrIniResvd/1
  HfaMotor_Runnable_sys/8(ActCfrModGroup_ActCfrModGroup_write) -> ActCfrModGroup_ActCfrModGroup/1
  HfaMotor_Runnable_sys/9(ActCfrModItem_ActCfrModItem_write) -> ActCfrModItem_ActCfrModItem/1
  HfaMotor_Runnable_sys/10(ActCfrModNewMod_ActCfrModNewMod_write) -> ActCfrModNewMod_ActCfrModNewMod/1
  HfaMotor_Runnable_sys/11(ActCfrModResvd1_ActCfrModResvd1_write) -> ActCfrModResvd1_ActCfrModResvd1/1
  HfaMotor_Runnable_sys/12(ActCfrModResvd2_ActCfrModResvd2_write) -> ActCfrModResvd2_ActCfrModResvd2/1
  HfaMotor_Runnable_sys/13(ActCfrPrgDiret_ActCfrPrgDiret_write) -> ActCfrPrgDiret_ActCfrPrgDiret/1
  HfaMotor_Runnable_sys/14(ActCfrPrgGroup_ActCfrPrgGroup_write) -> ActCfrPrgGroup_ActCfrPrgGroup/1
  HfaMotor_Runnable_sys/15(ActCfrPrgItem_ActCfrPrgItem_write) -> ActCfrPrgItem_ActCfrPrgItem/1
  HfaMotor_Runnable_sys/16(ActCfrPrgPrgGroup_ActCfrPrgPrgGroup_write) -> ActCfrPrgPrgGroup_ActCfrPrgPrgGroup/1
  HfaMotor_Runnable_sys/17(ActCfrPrgPrgItem_ActCfrPrgPrgItem_write) -> ActCfrPrgPrgItem_ActCfrPrgPrgItem/1
  HfaMotor_Runnable_sys/18(ActCfrPrgRef_ActCfrPrgRef_write) -> ActCfrPrgRef_ActCfrPrgRef/1
  HfaMotor_Runnable_sys/19(ActCfrPrgSafety_ActCfrPrgSafety_write) -> ActCfrPrgSafety_ActCfrPrgSafety/1
  HfaMotor_Runnable_sys/20(ActCfrPrgSafetyEnable_ActCfrPrgSafetyEnable_write) -> ActCfrPrgSafetyEnable_ActCfrPrgSafetyEnable/1
  HfaMotor_Runnable_sys/21(ActCfrSetAutoSpd_ActCfrSetAutoSpd_write) -> ActCfrSetAutoSpd_ActCfrSetAutoSpd/1
  HfaMotor_Runnable_sys/22(ActCfrSetFPos_ActCfrSetFPos_write) -> ActCfrSetFPos_ActCfrSetFPos/1
  HfaMotor_Runnable_sys/23(ActCfrSetGroup_ActCfrSetGroup_write) -> ActCfrSetGroup_ActCfrSetGroup/1
  HfaMotor_Runnable_sys/24(ActCfrSetItem_ActCfrSetItem_write) -> ActCfrSetItem_ActCfrSetItem/1
  HfaMotor_Runnable_sys/25(ActCfrSetLowNoise_ActCfrSetLowNoise_write) -> ActCfrSetLowNoise_ActCfrSetLowNoise/1
  HfaMotor_Runnable_sys/26(ActCfrSetSpd_ActCfrSetSpd_write) -> ActCfrSetSpd_ActCfrSetSpd/1
  HfaMotor_Runnable_sys/27(ActCfrSetStallDet_ActCfrSetStallDet_write) -> ActCfrSetStallDet_ActCfrSetStallDet/1
  HfaMotor_Runnable_sys/28(ActCfrSetStopPhs_ActCfrSetStopPhs_write) -> ActCfrSetStopPhs_ActCfrSetStopPhs/1
  HfaMotor_Runnable_sys/29(ActDfrIdeGroup_ActDfrIdeGroup_write) -> ActDfrIdeGroup_ActDfrIdeGroup/1
  HfaMotor_Runnable_sys/30(ActDfrIdeItem_ActDfrIdeItem_write) -> ActDfrIdeItem_ActDfrIdeItem/1
  HfaMotor_Runnable_sys/31(ActDfrIdeResvd_ActDfrIdeResvd_write) -> ActDfrIdeResvd_ActDfrIdeResvd/1
  HfaMotor_Runnable_sys/32(ActDfrPrgGroup_ActDfrPrgGroup_write) -> ActDfrPrgGroup_ActDfrPrgGroup/1
  HfaMotor_Runnable_sys/33(ActDfrPrgItem_ActDfrPrgItem_write) -> ActDfrPrgItem_ActDfrPrgItem/1
  HfaMotor_Runnable_sys/34(ActDfrPrgResvd_ActDfrPrgResvd_write) -> ActDfrPrgResvd_ActDfrPrgResvd/1
  HfaMotor_Runnable_sys/35(ActDfrStaGroup_ActDfrStaGroup_write) -> ActDfrStaGroup_ActDfrStaGroup/1
  HfaMotor_Runnable_sys/36(ActDfrStaItem_ActDfrStaItem_write) -> ActDfrStaItem_ActDfrStaItem/1
  HfaMotor_Runnable_sys/37(ActDfrStaResvd_ActDfrStaResvd_write) -> ActDfrStaResvd_ActDfrStaResvd/1
  HfaMotor_Runnable_sys/38(CcmHfaMasterReqB0Lin4_CcmHfaMasterReqB0Lin4_write) -> CcmHfaMasterReqB0Lin4_CcmHfaMasterReqB0Lin4/1
  HfaMotor_Runnable_sys/39(CcmHfaMasterReqB1Lin4_CcmHfaMasterReqB1Lin4_write) -> CcmHfaMasterReqB1Lin4_CcmHfaMasterReqB1Lin4/1
  HfaMotor_Runnable_sys/40(CcmHfaMasterReqB2Lin4_CcmHfaMasterReqB2Lin4_write) -> CcmHfaMasterReqB2Lin4_CcmHfaMasterReqB2Lin4/1
  HfaMotor_Runnable_sys/41(CcmHfaMasterReqB3Lin4_CcmHfaMasterReqB3Lin4_write) -> CcmHfaMasterReqB3Lin4_CcmHfaMasterReqB3Lin4/1
  HfaMotor_Runnable_sys/42(CcmHfaMasterReqB4Lin4_CcmHfaMasterReqB3Lin4_write) -> CcmHfaMasterReqB4Lin4_CcmHfaMasterReqB3Lin4/1
  HfaMotor_Runnable_sys/43(CcmHfaMasterReqB5Lin4_CcmHfaMasterReqB5Lin4_write) -> CcmHfaMasterReqB5Lin4_CcmHfaMasterReqB5Lin4/1
  HfaMotor_Runnable_sys/44(CcmHfaMasterReqB6Lin4_CcmHfaMasterReqB6Lin4_write) -> CcmHfaMasterReqB6Lin4_CcmHfaMasterReqB6Lin4/1
  HfaMotor_Runnable_sys/45(CcmHfaMasterReqB7Lin4_CcmHfaMasterReqB7Lin4_write) -> CcmHfaMasterReqB7Lin4_CcmHfaMasterReqB7Lin4/1
  HfaMotor_Runnable_sys/46(Lin1HfaActvReq_Lin1HfaActvReq_write) -> Lin1HfaActvReq_Lin1HfaActvReq/1
  HfaMotor_Runnable_sys/47(ReHvacMotAdr_ReHvacMotAdr_write) -> ReHvacMotAdr_ReHvacMotAdr/1
  HfaMotor_Runnable_sys/48(ReHvacMotBlockCmd_ReHvacMotBlockCmd_write) -> ReHvacMotBlockCmd_ReHvacMotBlockCmd/1
  HfaMotor_Runnable_sys/49(ReHvacMotClearReq_ReHvacMotClearReq_write) -> ReHvacMotClearReq_ReHvacMotClearReq/1
  HfaMotor_Runnable_sys/50(ReHvacMotCoilPwrOn_ReHvacMotCoilPwrOn_write) -> ReHvacMotCoilPwrOn_ReHvacMotCoilPwrOn/1
  HfaMotor_Runnable_sys/51(ReHvacMotDir_ReHvacMotDir_write) -> ReHvacMotDir_ReHvacMotDir/1
  HfaMotor_Runnable_sys/52(ReHvacMotMode_ReHvacMotMode_write) -> ReHvacMotMode_ReHvacMotMode/1
  HfaMotor_Runnable_sys/53(ReHvacMotPsonAvl_ReHvacMotPsonAvl_write) -> ReHvacMotPsonAvl_ReHvacMotPsonAvl/1
  HfaMotor_Runnable_sys/54(ReHvacMotSaveData_ReHvacMotSaveData_write) -> ReHvacMotSaveData_ReHvacMotSaveData/1
  HfaMotor_Runnable_sys/55(ReHvacMotSpdSet_ReHvacMotSpdSet_write) -> ReHvacMotSpdSet_ReHvacMotSpdSet/1
  HfaMotor_Runnable_sys/56(ReHvacMotStrtPson_ReHvacMotStrtPson_write) -> ReHvacMotStrtPson_ReHvacMotStrtPson/1
  HfaMotor_Runnable_sys/57(ReHvacMotTargtPson_ReHvacMotTargtPson_write) -> ReHvacMotTargtPson_ReHvacMotTargtPson/1
  HfaMotor_Runnable_sys/58(ReHvacMotUrgcPson_ReHvacMotUrgcPson_write) -> ReHvacMotUrgcPson_ReHvacMotUrgcPson/1
  HfaMotor_Runnable_sys/59(ReHvacMotUrgcUnlck_ReHvacMotUrgcUnlck_write) -> ReHvacMotUrgcUnlck_ReHvacMotUrgcUnlck/1
  HfaMotor_Runnable_sys/60(RHFAScheTableReq_Val_write) -> RHFAScheTableReq_Val/1
  HfaMotor_Runnable_sys/61(WtrMotAdr_WtrMotAdr_write) -> WtrMotAdr_WtrMotAdr/1
  HfaMotor_Runnable_sys/62(WtrMotBlockCmd_WtrMotBlockCmd_write) -> WtrMotBlockCmd_WtrMotBlockCmd/1
  HfaMotor_Runnable_sys/63(WtrMotClearReq_WtrMotClearReq_write) -> WtrMotClearReq_WtrMotClearReq/1
  HfaMotor_Runnable_sys/64(WtrMotCoilPwrOn_WtrMotCoilPwrOn_write) -> WtrMotCoilPwrOn_WtrMotCoilPwrOn/1
  HfaMotor_Runnable_sys/65(WtrMotDir_WtrMotDir_write) -> WtrMotDir_WtrMotDir/1
  HfaMotor_Runnable_sys/66(WtrMotMode_WtrMotMode_write) -> WtrMotMode_WtrMotMode/1
  HfaMotor_Runnable_sys/67(WtrMotPsonAvl_WtrMotPsonAvl_write) -> WtrMotPsonAvl_WtrMotPsonAvl/1
  HfaMotor_Runnable_sys/68(WtrMotSaveData_WtrMotSaveData_write) -> WtrMotSaveData_WtrMotSaveData/1
  HfaMotor_Runnable_sys/69(WtrMotSpdSet_WtrMotSpdSet_write) -> WtrMotSpdSet_WtrMotSpdSet/1
  HfaMotor_Runnable_sys/70(WtrMotStrtPson_WtrMotStrtPson_write) -> WtrMotStrtPson_WtrMotStrtPson/1
  HfaMotor_Runnable_sys/71(WtrMotTargtPson_WtrMotTargtPson_write) -> WtrMotTargtPson_WtrMotTargtPson/1
  HfaMotor_Runnable_sys/72(WtrMotUrgcPson_WtrMotUrgcPson_write) -> WtrMotUrgcPson_WtrMotUrgcPson/1
  HfaMotor_Runnable_sys/73(WtrMotUrgcUnlck_WtrMotUrgcUnlck_write) -> WtrMotUrgcUnlck_WtrMotUrgcUnlck/1
  HfaMotor_Runnable_sys/74(HfaMotAdr_HfaMotAdr_write) -> HfaMotAdr_HfaMotAdr/1
  HfaMotor_Runnable_sys/75(HfaMotBlockCmd_HfaMotBlockCmd_write) -> HfaMotBlockCmd_HfaMotBlockCmd/1
  HfaMotor_Runnable_sys/76(HfaMotClearReq_HfaMotClearReq_write) -> HfaMotClearReq_HfaMotClearReq/1
  HfaMotor_Runnable_sys/77(HfaMotCoilPwrOn_HfaMotCoilPwrOn_write) -> HfaMotCoilPwrOn_HfaMotCoilPwrOn/1
  HfaMotor_Runnable_sys/78(HfaMotDir_HfaMotDir_write) -> HfaMotDir_HfaMotDir/1
  HfaMotor_Runnable_sys/79(HfaMotMode_HfaMotMode_write) -> HfaMotMode_HfaMotMode/1
  HfaMotor_Runnable_sys/80(HfaMotPsonAvl_HfaMotPsonAvl_write) -> HfaMotPsonAvl_HfaMotPsonAvl/1
  HfaMotor_Runnable_sys/81(HfaMotSaveData_HfaMotSaveData_write) -> HfaMotSaveData_HfaMotSaveData/1
  HfaMotor_Runnable_sys/82(HfaMotSpdSet_HfaMotSpdSet_write) -> HfaMotSpdSet_HfaMotSpdSet/1
  HfaMotor_Runnable_sys/83(HfaMotStrtPson_HfaMotStrtPson_write) -> HfaMotStrtPson_HfaMotStrtPson/1
  HfaMotor_Runnable_sys/84(HfaMotTargtPson_HfaMotTargtPson_write) -> HfaMotTargtPson_HfaMotTargtPson/1
  HfaMotor_Runnable_sys/85(HfaMotUrgcPson_HfaMotUrgcPson_write) -> HfaMotUrgcPson_HfaMotUrgcPson/1
  HfaMotor_Runnable_sys/86(HfaMotUrgcUnlck_HfaMotUrgcUnlck_write) -> HfaMotUrgcUnlck_HfaMotUrgcUnlck/1
  BswLifeCycle_LifeCycle/1 -> HfaMotor_Runnable_sys/452(BswLifeCycle_LifeCycle_read)
  SbcWakeupSta_SbcWakeupSta/1 -> [SbcWakeupSta_SbcWakeupSta_Tag]
  [SbcWakeupSta_SbcWakeupSta_Tag] -> HfaMotor_Init/2(SbcWakeupSta_SbcWakeupSta_read)
  [SbcWakeupSta_SbcWakeupSta_Tag] -> HfaMotor_Runnable_sys/37(SbcWakeupSta_SbcWakeupSta_read)
  SbcPwrOn_SbcPwrOn/1 -> [SbcPwrOn_SbcPwrOn_Tag]
  [SbcPwrOn_SbcPwrOn_Tag] -> HfaMotor_Init/1(SbcPwrOn_SbcPwrOn_read)
  [SbcPwrOn_SbcPwrOn_Tag] -> HfaMotor_Runnable_sys/36(SbcPwrOn_SbcPwrOn_read)
  CCMHfaMotor_HfaMotMode/1 -> CCMHfaMotor_HfaMotMode_sys/Trigger
  CCMHfaMotor_ReHvacMotMode/1 -> CCMHfaMotor_ReHvacMotMode_sys/Trigger
  CCMHfaMotor_WtrMotMode/1 -> CCMHfaMotor_WtrMotMode_sys/Trigger
  HfaMotor_50msRunnable/1 -> HfaMotor_50msRunnable_sys/Trigger
  HfaMotor_Runnable/1 -> HfaMotor_Runnable_sys/Trigger
